simulink model "MemMgrForClima"  (R2022b)
settings: Solver=FixedStepDiscrete  StartTime=0.0  StopTime=10.0  FixedStep=0.1  RelTol=1e-3
model workspace (MAT data in the model file):
  MemMgrForClimaNvArray = Simulink.Signal
  MemMgrForClimaNvArray.DataType = 'UInt8'
  MemMgrForClimaNvArray.Dimensions = [254 1]
  MemMgrForClimaNvArray.DimensionsMode = 'auto'
  MemMgrForClimaNvArray.Complexity = 'real'
  MemMgrForClimaNvArray.SampleTime = -1
  MemMgrForClimaNvArray.SamplingMode = 'auto'
  MemMgrForClimaNvArray.InitialValue = 'MemMgrForClimaNvInit'
  MemMgrForClimaNvInit = Simulink.Parameter
  MemMgrForClimaNvInit.Value = [0; 0; 0; 0; 0; 0; 0; 0; 0; 0; 0; 0; 0; 0; 0; 0; 0; 0; 0; 0; 0; 0; 0; 0; 0; 0; 0; 0; 0; 0; 0; 0; 0; 0; 0; 0; 0; 0; 0; 0; 0; 0; 0; 0; 0; 0; 0; 0; 0; 0; 0; 0; 0; 0; 0; 0; 0; 0; 0; 0; 0; 0; 0; 0; 0; 0; 0; 0; 0; 0; 0; 0; 0; 0; 0; 0; 0; 0; 0; 0; 0; 0; 0; 0; 0; 0; 0; 0; 0; 0; 0; 0; 0; 0; 0; 0; 0; 0; 0; 0; 0; 0; 0; 0; 0; 0; 0; 0; 0; 0; 0; 0; 0; 0; 0; 0; 0; 0; 0; 0; 0; 0; 0; 0; 0; 0; 0; 0; 0; 0; 0; 0; 0; 0; 0; 0; 0; 0; 0; 0; 0; 0; 0; 0; 0; 0; 0; 0; 0; 0; 0; 0; 0; 0; 0; 0; 0; 0; 0; 0; 0; 0; 0; 0; 0; 0; 0; 0; 0; 0; 0; 0; 0; 0; 0; 0; 0; 0; 0; 0; 0; 0; 0; 0; 0; 0; 0; 0; 0; 0; 0; 0; 0; 0; 0; 0; 0; 0; 0; 0; 0; 0; 0; 0; 0; 0; 0; 0; 0; 0; 0; 0; 0; 0; 0; 0; 0; 0; 0; 0; 0; 0; 0; 0; 0; 0; 0; 0; 0; 0; 0; 0; 0; 0; 0; 0; 0; 0; 0; 0; 0; 0; 0; 0; 0; 0; 0; 0; 0; 0; 0; 0; 0; 0]
  MemMgrForClimaNvInit.DataType = 'UInt8'
  MemMgrForClimaNvInit.Complexity = 'real'
  MemMgrForClimaNvInit.Dimensions = [254 1]
(part 1 of 2)
settings Model: Creator=blue.ge  LastModifiedBy=blue.ge
settings DataIOCC: SaveFormat=StructureWithTime
settings OptimizationCC: DefaultParameterBehavior=Inlined  EnhancedBackFolding=on  PassReuseOutputArgsAs=Individual arguments  ZeroExternalMemoryAtStartup=off  ZeroInternalMemoryAtStartup=off  OptimizeBlockOrder=speed  DifferentSizesBufferReuse=on  OptimizationCustomize=off  EfficientTunableParamExpr=on
settings DebuggingCC: ParameterTunabilityLossMsg=error
settings ModelReferenceCC: ModelReferenceNumInstancesAllowed=Single
settings SFSimCC: MATLABDynamicMemAlloc=off
settings RTWCC: Description=AUTOSAR  SystemTargetFile=autosar.tlc  GenCodeOnly=on  TemplateMakefile=ert_default_tmf
settings CodeAppCC: ForceParamTrailComments=on  IgnoreCustomStorageClasses=off  OperatorAnnotations=on  SimulinkDataObjDesc=on  SFDataObjDesc=on  InsertBlockDesc=on
settings STFCustomTargetCC: TargetLangStandard=C99 (ISO)  MultiwordLength=256  ParMdlRefBuildCompliant=on  CompOptLevelCompliant=on  IncludeMdlTerminateFcn=off  CombineOutputUpdateFcns=on  SuppressErrorStatus=on  ERTCustomFileBanners=on  SupportNonFinite=off  SupportComplex=off  SupportContinuousTime=off  SupportNonInlinedSFcns=off  RemoveResetFunc=on  MATLABClassNameForMDSCustomization=Simulink.SoftwareTarget.ERTCustomization  AutosarCompliant=on  UseToolchainInfoCompliant=on  LookupTableObjectStructAxisOrder=2,1,3,4,...  LUTObjectStructOrderEvenSpacing=Size,Table,Breakpoints  SystemTargetFile=autosar.tlc
settings ERTTargetCC: TargetLangStandard=C99 (ISO)  ConcurrentExecutionCompliant=off  IncludeMdlTerminateFcn=off  SuppressErrorStatus=on  SupportNonFinite=off  SupportComplex=off  ModelStepFunctionPrototypeControlCompliant=off  CPPClassGenCompliant=off  AutosarCompliant=on  LookupTableObjectStructAxisOrder=2,1,3,4,...  LUTObjectStructOrderEvenSpacing=Size,Table,Breakpoints  InlinedParameterPlacement=NonHierarchical  ERTCustomFileTemplate=example_file_process.tlc

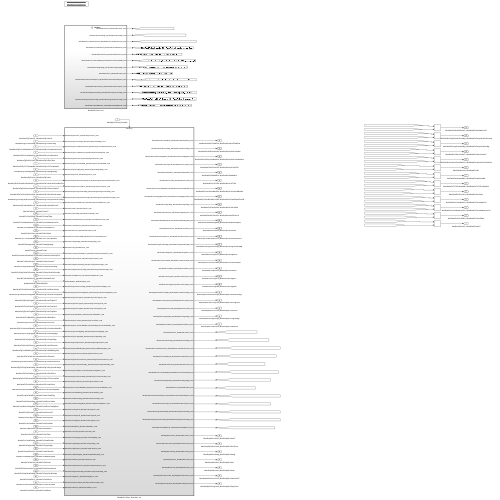
[thumbnail: Simulink's stored preview of the top-level diagram]
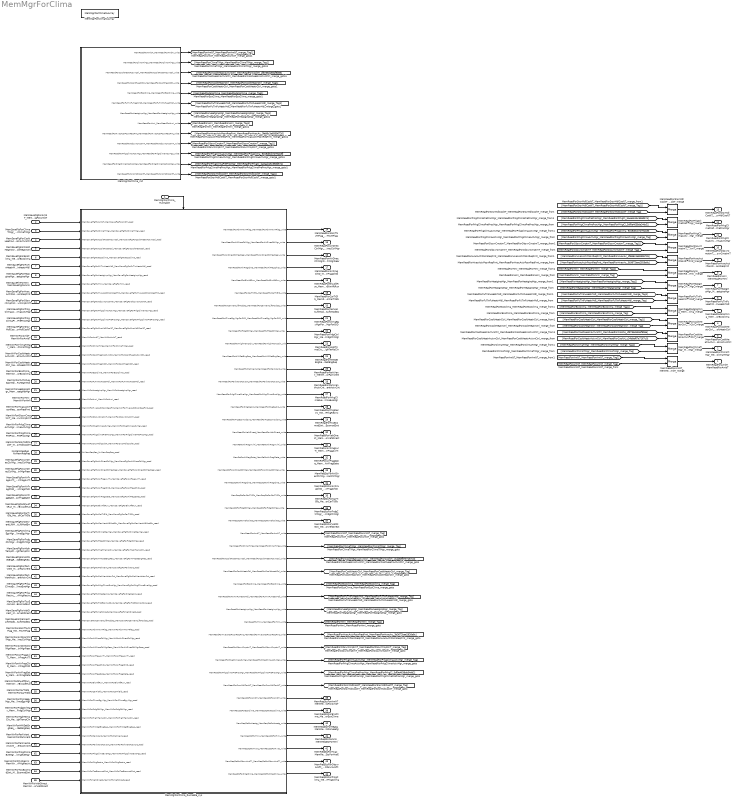
== system MemMgrForClima ==
  MemMgrForClima_Runnable : Inport  SampleTime=0.1  OutputFunctionCall=on
  MemSaveFlgForAmbT_MemSaveFlgForAmbT : Inport  Port=2  OutDataTypeStr=Boolean  PortDimensions=1
  MemSaveFlgForClimaTiMgr_MemSaveFlgForClimaTiMgr : Inport  Port=3  OutDataTypeStr=Boolean  PortDimensions=1
  MemSaveFlgForCooltHeatrActvnCtrl_MemSaveFlgForCooltHeatrActvnCtrl : Inport  Port=4  OutDataTypeStr=Boolean  PortDimensions=1
  MemSaveFlgForCooltHeatrCtrl_MemSaveFlgForCooltHeatrCtrl : Inport  Port=5  OutDataTypeStr=Boolean  PortDimensions=1
  MemSaveFlgForEcoClima_MemSaveFlgForEcoClima : Inport  Port=6  OutDataTypeStr=Boolean  PortDimensions=1
  MemSaveFlgForFuTInHeatrMdl_MemSaveFlgForFuTInHeatrMdl : Inport  Port=7  OutDataTypeStr=Boolean  PortDimensions=1
  MemSaveFlgForHeatgInpMgr_MemSaveFlgForHeatgInpMgr : Inport  Port=8  OutDataTypeStr=Boolean  PortDimensions=1
  MemSaveFlgForHmi_MemSaveFlgForHmi : Inport  Port=9  OutDataTypeStr=Boolean  PortDimensions=1
  MemSaveFlgForHvacActrPosnReqFrnt_MemSaveFlgForHvacActrPosnReqFrnt : Inport  Port=10  OutDataTypeStr=Boolean  PortDimensions=1
  MemSaveFlgForObsvrCmptmtT_MemSaveFlgForObsvrCmptmtT : Inport  Port=11  OutDataTypeStr=Boolean  PortDimensions=1
  MemSaveFlgForPrkgClimaActvMgr_MemSaveFlgForPrkgClimaActvMgr : Inport  Port=12  OutDataTypeStr=Boolean  PortDimensions=1
  MemSaveFlgForPrkgClimaPreProcMgr_MemSaveFlgForPrkgClimaPreProcMgr : Inport  Port=13  OutDataTypeStr=Boolean  PortDimensions=1
  MemSaveFlgForSnsrMdlCooltT_MemSaveFlgForSnsrMdlCooltT : Inport  Port=14  OutDataTypeStr=Boolean  PortDimensions=1
  MemWrForAmbT_MemWrForAmbT : Inport  Port=15  OutDataTypeStr=T30  PortDimensions=1
  MemWrForClimaTiMgr_MemWrForClimaTiMgr : Inport  Port=16  OutDataTypeStr=Bus: MemRecForClimaTiMgr  PortDimensions=1  BusOutputAsStruct=on
  MemWrForCooltHeatrActvnCtrl_MemWrForCooltHeatrActvnCtrl : Inport  Port=17  OutDataTypeStr=Bus: MemRecForCooltHeatrActvnCtrl  PortDimensions=1  BusOutputAsStruct=on
  MemWrForCooltHeatrCtrl_MemWrForCooltHeatrCtrl : Inport  Port=18  OutDataTypeStr=Bus: MemRecForCooltHeatrCtrl  PortDimensions=1  BusOutputAsStruct=on
  MemWrForEcoClima_MemWrForEcoClima : Inport  Port=19  OutDataTypeStr=Bus: MemWrForEcoClima  PortDimensions=1  BusOutputAsStruct=on
  MemWrForFuTInFuHeatrMdl_MemWrForFuTInFuHeatrMdl : Inport  Port=20  OutDataTypeStr=Bus: MemRecForFuTInFuHeatrMdl  PortDimensions=1  BusOutputAsStruct=on
  MemWrForHeatgInpMgr_MemWrForHeatgInpMgr : Inport  Port=21  OutDataTypeStr=Bus: MemRecForHeatgInpMgr  PortDimensions=1  BusOutputAsStruct=on
  MemWrForHmi_MemWrForHmi : Inport  Port=22  OutDataTypeStr=Bus: MemRecForHmi  PortDimensions=1  BusOutputAsStruct=on
  MemWrForHvacActrPosnReqFrnt_MemWrForHvacActrPosnReqFrnt : Inport  Port=23  OutDataTypeStr=Bus: MemRecForHvacActrPosnReqFrnt  PortDimensions=1  BusOutputAsStruct=on
  MemWrForObsvrCmptmT_MemWrForObsvrCmptmT : Inport  Port=24  OutDataTypeStr=Bus: MemRecForObsvrCmptmT  PortDimensions=1  BusOutputAsStruct=on
  MemWrForPrkgClimaActvMgr_MemWrForPrkgClimaActvMgr : Inport  Port=25  OutDataTypeStr=Bus: MemRecForPrkgClimaActvMgr  PortDimensions=1  BusOutputAsStruct=on
  MemWrForPrkgClimaPreProcMgr_MemWrForPrkgClimaPreProcMgr : Inport  Port=26  OutDataTypeStr=Bus: MemRecForPrkgClimaPreProcMgr  PortDimensions=1  BusOutputAsStruct=on
  MemWrForSnsrMdlCooltT_MemWrForSnsrMdlCooltT : Inport  Port=27  OutDataTypeStr=Bus: MemRecForSnsrMdlCooltT  PortDimensions=1  BusOutputAsStruct=on
  NvMemReqRes_NvMemReqRes : Inport  Port=28  OutDataTypeStr=Enum: NvMemReqRes  PortDimensions=1
  MemSaveFlgForAirDirecCtrlMgr_MemSaveFlgForAirDirecCtrlMgr : Inport  Port=29  OutDataTypeStr=Boolean  PortDimensions=1
  MemSaveFlgForAirDirecCtrlMgrRear_MemSaveFlgForAirDirecCtrlMgrRear : Inport  Port=30  OutDataTypeStr=Boolean  PortDimensions=1
  MemSaveFlgForAirFragAvlTi_MemSaveFlgForAirFragAvlTi : Inport  Port=31  OutDataTypeStr=Boolean  PortDimensions=1
  MemSaveFlgForAirFragChID_MemSaveFlgForAirFragChID : Inport  Port=32  OutDataTypeStr=Boolean  PortDimensions=1
  MemSaveFlgForAirFragDate_MemSaveFlgForAirFragDate : Inport  Port=33  OutDataTypeStr=Boolean  PortDimensions=1
  MemSaveFlgForBlwAftRun_MemSaveFlgForBlwAftRun : Inport  Port=34  OutDataTypeStr=Boolean  PortDimensions=1
  MemSaveFlgForCarTiGlb_MemSaveFlgForCarTiGlb : Inport  Port=35  OutDataTypeStr=Boolean  PortDimensions=1
  MemSaveFlgForCenVentLiftModErr_MemSaveFlgForCenVentLiftModErr : Inport  Port=36  OutDataTypeStr=Boolean  PortDimensions=1
  MemSaveFlgForClimaEgyMgr_MemSaveFlgForClimaEgyMgr : Inport  Port=37  OutDataTypeStr=Boolean  PortDimensions=1
  MemSaveFlgForFrdgCtrlMgr_MemSaveFlgForFrdgCtrlMgr : Inport  Port=38  OutDataTypeStr=Boolean  PortDimensions=1
  MemSaveFlgForHighTempCln_MemSaveFlgForHighTempCln : Inport  Port=39  OutDataTypeStr=Boolean  PortDimensions=1
  MemSaveFlgForHMISetEngReq_MemSaveFlgForHMISetEngReq : Inport  Port=40  OutDataTypeStr=Boolean  PortDimensions=1
  MemSaveFlgForParkVent_MemSaveFlgForParkVent : Inport  Port=41  OutDataTypeStr=Boolean  PortDimensions=1
  MemSaveFlgForParkVentActvCnt_MemSaveFlgForParkVentActvCnt : Inport  Port=42  OutDataTypeStr=Boolean  PortDimensions=1
  MemSaveFlgForPrkgClimaEveMgr_MemSaveFlgForPrkgClimaEveMgr : Inport  Port=43  OutDataTypeStr=Boolean  PortDimensions=1
  MemSaveFlgForPrkgRecirc_MemSaveFlgForPrkgRecirc : Inport  Port=44  OutDataTypeStr=Boolean  PortDimensions=1
  MemSaveFlgForTcsEcomodSwt_MemSaveFlgForTcsEcomodSwt : Inport  Port=45  OutDataTypeStr=Boolean  PortDimensions=1
  MemSaveFlgForVehDirect_MemSaveFlgForVehDirect : Inport  Port=46  OutDataTypeStr=Boolean  PortDimensions=1
  MemSaveForCenVentLiftModSts_MemSaveForCenVentLiftModSts : Inport  Port=47  OutDataTypeStr=Bus: MemSaveLiftMod  PortDimensions=1  BusOutputAsStruct=on
  MemWrForAbmTNvMFlag_MemWrForAbmTNvMFlag : Inport  Port=48  OutDataTypeStr=Boolean  PortDimensions=1
  MemWrForAirDirecCtrlMgr_MemWrForAirDirecCtrlMgr : Inport  Port=49  OutDataTypeStr=Bus: MemRecForAirDirecCtrlMgr  PortDimensions=1  BusOutputAsStruct=on
  MemWrForAirDirecCtrlMgrRear_MemWrForAirDirecCtrlMgrRear : Inport  Port=50  OutDataTypeStr=Bus: MemRecForAirDirecCtrlMgrRear  PortDimensions=1  BusOutputAsStruct=on
  MemWrForAirFragAvlTi_MemWrForAirFragAvlTi : Inport  Port=51  OutDataTypeStr=Bus: MemReqForAirFragAvlTi  PortDimensions=1  BusOutputAsStruct=on
  MemWrForAirFragChID_MemWrForAirFragChID : Inport  Port=52  OutDataTypeStr=Bus: MemReqForAirFragChID  PortDimensions=1  BusOutputAsStruct=on
  MemWrForAirFragDate_MemWrForAirFragDate : Inport  Port=53  OutDataTypeStr=Bus: MemWrForAirFragDate  PortDimensions=1  BusOutputAsStruct=on
  MemWrForBlwAftRun_MemWrForBlwAftRun : Inport  Port=54  OutDataTypeStr=Bus: MemRecForBlwAftRun  PortDimensions=1  BusOutputAsStruct=on
  MemWrForCarTiGlb_MemWrForCarTiGlb : Inport  Port=55  OutDataTypeStr=CarTiGlb  PortDimensions=1
  MemWrForClimaEgyMgr_MemWrForClimaEgyMgr : Inport  Port=56  OutDataTypeStr=Bus: MemRecForClimaEgyMgr  PortDimensions=1  BusOutputAsStruct=on
  MemWrForFrdgCtrlMgr_MemWrForFrdgCtrlMgr : Inport  Port=57  OutDataTypeStr=Bus: MemRecForFrdgCtrlMgr  PortDimensions=1  BusOutputAsStruct=on
  MemWrForHighTempCln_MemWrForHighTempCln : Inport  Port=58  OutDataTypeStr=Bus: MemRecForHighTempCln  PortDimensions=1  BusOutputAsStruct=on
  MemWrForHMISetEngReq_MemWrForHMISetEngReq : Inport  Port=59  OutDataTypeStr=Bus: MemRecForHMISetEngReq  PortDimensions=1  BusOutputAsStruct=on
  MemWrForParkVent_MemWrForParkVent : Inport  Port=60  OutDataTypeStr=Bus: MemRecForParkVent  PortDimensions=1  BusOutputAsStruct=on
  MemWrForParkVentActvCnt_MemWrForParkVentActvCnt : Inport  Port=61  OutDataTypeStr=Bus: MemRecForParkVentCnt  PortDimensions=1  BusOutputAsStruct=on
  MemWrForPrkgClimaEveMgr_MemWrForPrkgClimaEveMgr : Inport  Port=62  OutDataTypeStr=Bus: MemRecForPrkgClimaEveMgr  PortDimensions=1  BusOutputAsStruct=on
  MemWrForPrkgRecirc_MemWrForPrkgRecirc : Inport  Port=63  OutDataTypeStr=UInt8  PortDimensions=1
  MemWrForTcsEcomodSwt_MemWrForTcsEcomodSwt : Inport  Port=64  OutDataTypeStr=Boolean  PortDimensions=1
  MemWrForVehDirect_MemWrForVehDirect : Inport  Port=65  OutDataTypeStr=VCCVehDirect  PortDimensions=1
  MemMgrForClimaNvArray : DataStoreMemory  DataStoreName=MemMgrForClimaNvArray  InitialValue=MemMgrForClimaNvInit  ShowAdditionalParam=off  Dimensions=254  StateMustResolveToSignalObject=on
  MemMgrForClima_Init : Subsystem
  MemMgrForClima_Runnable_sys : Subsystem
  MemReadForAmbT_MemReadForAmbT_merge : Merge
  MemReadForClimaTiMgr_MemReadForClimaTiMgr_merge : Merge
  MemReadForCooltHeatrActvnCtrl_MemReadForCooltHeatrActvnCtrl_merge : Merge
  MemReadForCooltHeatrCtrl_MemReadForCooltHeatrCtrl_merge : Merge
  MemReadForEcoClima_MemReadForEcoClima_merge : Merge
  MemReadForFuTInFuHeatrMdl_MemReadForFuTInFuHeatrMdl_merge : Merge
  MemReadForHeatgInpMgr_MemReadForHeatgInpMgr_merge : Merge
  MemReadForHmi_MemReadForHmi_merge : Merge
  MemReadForHvacActrPosnReqFrnt_MemReadForHvacActrPosnReqFrnt_merge : Merge
  MemReadForObsvrCmptmT_MemReadForObsvrCmptmT_merge : Merge
  MemReadForPrkgClimaActvMgr_MemReadForPrkgClimaActvMgr_merge : Merge
  MemReadForPrkgClimaPreProcMgr_MemReadForPrkgClimaPreProcMgr_merge : Merge
  MemReadForSnsrMdlCooltT_MemReadForSnsrMdlCooltT_merge : Merge
  MemReadForAmbT_MemReadForAmbT : Outport  OutDataTypeStr=T30  PortDimensions=1
  MemReadForClimaTiMgr_MemReadForClimaTiMgr : Outport  Port=2  OutDataTypeStr=Bus: MemRecForClimaTiMgr  PortDimensions=1  BusOutputAsStruct=on
  MemReadForCooltHeatrActvnCtrl_MemReadForCooltHeatrActvnCtrl : Outport  Port=3  OutDataTypeStr=Bus: MemRecForCooltHeatrActvnCtrl  PortDimensions=1  BusOutputAsStruct=on
  MemReadForCooltHeatrCtrl_MemReadForCooltHeatrCtrl : Outport  Port=4  OutDataTypeStr=Bus: MemRecForCooltHeatrCtrl  PortDimensions=1  BusOutputAsStruct=on
  MemReadForEcoClima_MemReadForEcoClima : Outport  Port=5  OutDataTypeStr=Bus: MemWrForEcoClima  PortDimensions=1  BusOutputAsStruct=on
  MemReadForFuTInFuHeatrMdl_MemReadForFuTInFuHeatrMdl : Outport  Port=6  OutDataTypeStr=Bus: MemRecForFuTInFuHeatrMdl  PortDimensions=1  BusOutputAsStruct=on
  MemReadForHeatgInpMgr_MemReadForHeatgInpMgr : Outport  Port=7  OutDataTypeStr=Bus: MemRecForHeatgInpMgr  PortDimensions=1  BusOutputAsStruct=on
  MemReadForHmi_MemReadForHmi : Outport  Port=8  OutDataTypeStr=Bus: MemRecForHmi  PortDimensions=1  BusOutputAsStruct=on
  MemReadForHvacActrPosnReqFrnt_MemReadForHvacActrPosnReqFrnt : Outport  Port=9  OutDataTypeStr=Bus: MemRecForHvacActrPosnReqFrnt  PortDimensions=1  BusOutputAsStruct=on
  MemReadForObsvrCmptmT_MemReadForObsvrCmptmT : Outport  Port=10  OutDataTypeStr=Bus: MemRecForObsvrCmptmT  PortDimensions=1  BusOutputAsStruct=on
  MemReadForPrkgClimaActvMgr_MemReadForPrkgClimaActvMgr : Outport  Port=11  OutDataTypeStr=Bus: MemRecForPrkgClimaActvMgr  PortDimensions=1  BusOutputAsStruct=on
  MemReadForPrkgClimaPreProcMgr_MemReadForPrkgClimaPreProcMgr : Outport  Port=12  OutDataTypeStr=Bus: MemRecForPrkgClimaPreProcMgr  PortDimensions=1  BusOutputAsStruct=on
  MemReadForSnsrMdlCooltT_MemReadForSnsrMdlCooltT : Outport  Port=13  OutDataTypeStr=Bus: MemRecForSnsrMdlCooltT  PortDimensions=1  BusOutputAsStruct=on
  MemReadForAbmTNvMFlag_MemReadForAbmTNvMFlag : Outport  Port=14  OutDataTypeStr=Boolean  PortDimensions=1
  MemReadForAirDirecCtrlMgr_MemReadForAirDirecCtrlMgr : Outport  Port=15  OutDataTypeStr=Bus: MemRecForAirDirecCtrlMgr  PortDimensions=1  BusOutputAsStruct=on
  MemReadForAirDirecCtrlMgrRear_MemReadForAirDirecCtrlMgrRear : Outport  Port=16  OutDataTypeStr=Bus: MemRecForAirDirecCtrlMgrRear  PortDimensions=1  BusOutputAsStruct=on
  MemReadForAirFragChID_MemReadForAirFragChID : Outport  Port=17  OutDataTypeStr=Bus: MemReqForAirFragChID  PortDimensions=1  BusOutputAsStruct=on
  MemReadForBlwAftRun_MemReadForBlwAftRun : Outport  Port=18  OutDataTypeStr=Bus: MemRecForBlwAftRun  PortDimensions=1  BusOutputAsStruct=on
  MemReadForCarTiGlb_MemReadForCarTiGlb : Outport  Port=19  OutDataTypeStr=CarTiGlb  PortDimensions=1
  MemReadForCenVentLiftModSts_MemReadForCenVentLiftModSts : Outport  Port=20  OutDataTypeStr=Bus: MemSaveLiftMod  PortDimensions=1  BusOutputAsStruct=on
  MemReadForClimaEgyMgrForDID_MemReadForClimaEgyMgrForDID : Outport  Port=21  OutDataTypeStr=Bus: MemRecForClimaEgyMgr  PortDimensions=1  BusOutputAsStruct=on
  MemReadForFrdgCtrlMgr_MemReadForFrdgCtrlMgr : Outport  Port=22  OutDataTypeStr=Bus: MemRecForFrdgCtrlMgr  PortDimensions=1  BusOutputAsStruct=on
  MemReadForHighTempCln_MemReadForHighTempCln : Outport  Port=23  OutDataTypeStr=Bus: MemRecForHighTempCln  PortDimensions=1  BusOutputAsStruct=on
  MemReadForHMISetEngReq_MemReadForHMISetEngReq : Outport  Port=24  OutDataTypeStr=Bus: MemRecForHMISetEngReq  PortDimensions=1  BusOutputAsStruct=on
  MemReadForParkVent_MemReadForParkVent : Outport  Port=25  OutDataTypeStr=Bus: MemRecForParkVent  PortDimensions=1  BusOutputAsStruct=on
  MemReadForParkVentActvCnt_MemReadForParkVentActvCnt : Outport  Port=26  OutDataTypeStr=Bus: MemRecForParkVentCnt  PortDimensions=1  BusOutputAsStruct=on
  MemReadForPrkgClimaEveMgr_MemReadForPrkgClimaEveMgr : Outport  Port=27  OutDataTypeStr=Bus: MemRecForPrkgClimaEveMgr  PortDimensions=1  BusOutputAsStruct=on
  MemReadForPrkgRecirc_MemReadForPrkgRecirc : Outport  Port=28  OutDataTypeStr=UInt8  PortDimensions=1
  MemReadForTcsEcomodSwt_MemReadForTcsEcomodSwt : Outport  Port=29  OutDataTypeStr=Boolean  PortDimensions=1
  MemReadForVehDirect_MemReadForVehDirect : Outport  Port=30  OutDataTypeStr=VCCVehDirect  PortDimensions=1
  MemReForAirFragAvlTi_MemReForAirFragAvlTi : Outport  Port=31  OutDataTypeStr=Bus: MemReqForAirFragAvlTi  PortDimensions=1  BusOutputAsStruct=on
  MemReForAirFragDate_MemReForAirFragDate : Outport  Port=32  OutDataTypeStr=Bus: MemWrForAirFragDate  PortDimensions=1  BusOutputAsStruct=on
  MemReqStsForAirDirecCtrlMgr_MemReqStsForAirDirecCtrlMgr : Outport  Port=33  OutDataTypeStr=Enum: MemReqSts  PortDimensions=1
  MemReqStsForAirFragChID_MemReqStsForAirFragChID : Outport  Port=34  OutDataTypeStr=Enum: MemReqSts  PortDimensions=1
  MemReqStsForCarTiGlb_MemReqStsForCarTiGlb : Outport  Port=35  OutDataTypeStr=Enum: MemReqSts  PortDimensions=1
  MemReqStsForFrdgCtrlMgr_MemReqStsForFrdgCtrlMgr : Outport  Port=36  OutDataTypeStr=Enum: MemReqSts  PortDimensions=1
  MemReqStsForVehDirect_MemReqStsForVehDirect : Outport  Port=37  OutDataTypeStr=Enum: MemReqSts  PortDimensions=1
  MemReqStsForAmbT_MemReqStsForAmbT : Outport  Port=38  OutDataTypeStr=Enum: MemReqSts  PortDimensions=1
  MemReqStsForEcoClima_MemReqStsForEcoClima : Outport  Port=39  OutDataTypeStr=Enum: MemReqSts  PortDimensions=1
  MemReqStsForHeatg_MemReqStsForHeatg : Outport  Port=40  OutDataTypeStr=Enum: MemReqSts  PortDimensions=1
  MemReqStsForHmi_MemReqStsForHmi : Outport  Port=41  OutDataTypeStr=Enum: MemReqSts  PortDimensions=1
  MemReqStsForHvac_MemReqStsForHvac : Outport  Port=42  OutDataTypeStr=Enum: MemReqSts  PortDimensions=1
  MemReqStsForObsvrAndTi_MemReqStsForObsvrAndTi : Outport  Port=43  OutDataTypeStr=Enum: MemReqSts  PortDimensions=1
  MemReqStsForPrkgClima_MemReqStsForPrkgClima : Outport  Port=44  OutDataTypeStr=Enum: MemReqSts  PortDimensions=1
  wires:
  MemSaveFlgForAmbT_MemSaveFlgForAmbT/1 -> MemMgrForClima_Runnable_sys/1(MemSaveFlgForAmbT_MemSaveFlgForAmbT_read)
  MemSaveFlgForClimaTiMgr_MemSaveFlgForClimaTiMgr/1 -> MemMgrForClima_Runnable_sys/2(MemSaveFlgForClimaTiMgr_MemSaveFlgForClimaTiMgr_read)
  MemSaveFlgForCooltHeatrActvnCtrl_MemSaveFlgForCooltHeatrActvnCtrl/1 -> MemMgrForClima_Runnable_sys/3(MemSaveFlgForCooltHeatrActvnCtrl_MemSaveFlgForCooltHeatrActvnCtrl_read)
  MemSaveFlgForCooltHeatrCtrl_MemSaveFlgForCooltHeatrCtrl/1 -> MemMgrForClima_Runnable_sys/4(MemSaveFlgForCooltHeatrCtrl_MemSaveFlgForCooltHeatrCtrl_read)
  MemSaveFlgForEcoClima_MemSaveFlgForEcoClima/1 -> MemMgrForClima_Runnable_sys/5(MemSaveFlgForEcoClima_MemSaveFlgForEcoClima_read)
  MemSaveFlgForFuTInHeatrMdl_MemSaveFlgForFuTInHeatrMdl/1 -> MemMgrForClima_Runnable_sys/6(MemSaveFlgForFuTInHeatrMdl_MemSaveFlgForFuTInHeatrMdl_read)
  MemSaveFlgForHeatgInpMgr_MemSaveFlgForHeatgInpMgr/1 -> MemMgrForClima_Runnable_sys/7(MemSaveFlgForHeatgInpMgr_MemSaveFlgForHeatgInpMgr_read)
  MemSaveFlgForHmi_MemSaveFlgForHmi/1 -> MemMgrForClima_Runnable_sys/8(MemSaveFlgForHmi_MemSaveFlgForHmi_read)
  MemSaveFlgForHvacActrPosnReqFrnt_MemSaveFlgForHvacActrPosnReqFrnt/1 -> MemMgrForClima_Runnable_sys/9(MemSaveFlgForHvacActrPosnReqFrnt_MemSaveFlgForHvacActrPosnReqFrnt_read)
  MemSaveFlgForObsvrCmptmtT_MemSaveFlgForObsvrCmptmtT/1 -> MemMgrForClima_Runnable_sys/10(MemSaveFlgForObsvrCmptmtT_MemSaveFlgForObsvrCmptmtT_read)
  MemSaveFlgForPrkgClimaActvMgr_MemSaveFlgForPrkgClimaActvMgr/1 -> MemMgrForClima_Runnable_sys/11(MemSaveFlgForPrkgClimaActvMgr_MemSaveFlgForPrkgClimaActvMgr_read)
  MemSaveFlgForPrkgClimaPreProcMgr_MemSaveFlgForPrkgClimaPreProcMgr/1 -> MemMgrForClima_Runnable_sys/12(MemSaveFlgForPrkgClimaPreProcMgr_MemSaveFlgForPrkgClimaPreProcMgr_read)
  MemSaveFlgForSnsrMdlCooltT_MemSaveFlgForSnsrMdlCooltT/1 -> MemMgrForClima_Runnable_sys/13(MemSaveFlgForSnsrMdlCooltT_MemSaveFlgForSnsrMdlCooltT_read)
  MemWrForAmbT_MemWrForAmbT/1 -> MemMgrForClima_Runnable_sys/14(MemWrForAmbT_MemWrForAmbT_read)
  MemWrForClimaTiMgr_MemWrForClimaTiMgr/1 -> MemMgrForClima_Runnable_sys/15(MemWrForClimaTiMgr_MemWrForClimaTiMgr_read)
  MemWrForCooltHeatrActvnCtrl_MemWrForCooltHeatrActvnCtrl/1 -> MemMgrForClima_Runnable_sys/16(MemWrForCooltHeatrActvnCtrl_MemWrForCooltHeatrActvnCtrl_read)
  MemWrForCooltHeatrCtrl_MemWrForCooltHeatrCtrl/1 -> MemMgrForClima_Runnable_sys/17(MemWrForCooltHeatrCtrl_MemWrForCooltHeatrCtrl_read)
  MemWrForEcoClima_MemWrForEcoClima/1 -> MemMgrForClima_Runnable_sys/18(MemWrForEcoClima_MemWrForEcoClima_read)
  MemWrForFuTInFuHeatrMdl_MemWrForFuTInFuHeatrMdl/1 -> MemMgrForClima_Runnable_sys/19(MemWrForFuTInFuHeatrMdl_MemWrForFuTInFuHeatrMdl_read)
  MemWrForHeatgInpMgr_MemWrForHeatgInpMgr/1 -> MemMgrForClima_Runnable_sys/20(MemWrForHeatgInpMgr_MemWrForHeatgInpMgr_read)
  MemWrForHmi_MemWrForHmi/1 -> MemMgrForClima_Runnable_sys/21(MemWrForHmi_MemWrForHmi_read)
  MemWrForHvacActrPosnReqFrnt_MemWrForHvacActrPosnReqFrnt/1 -> MemMgrForClima_Runnable_sys/22(MemWrForHvacActrPosnReqFrnt_MemWrForHvacActrPosnReqFrnt_read)
  MemWrForObsvrCmptmT_MemWrForObsvrCmptmT/1 -> MemMgrForClima_Runnable_sys/23(MemWrForObsvrCmptmT_MemWrForObsvrCmptmT_read)
  MemWrForPrkgClimaActvMgr_MemWrForPrkgClimaActvMgr/1 -> MemMgrForClima_Runnable_sys/24(MemWrForPrkgClimaActvMgr_MemWrForPrkgClimaActvMgr_read)
  MemWrForPrkgClimaPreProcMgr_MemWrForPrkgClimaPreProcMgr/1 -> MemMgrForClima_Runnable_sys/25(MemWrForPrkgClimaPreProcMgr_MemWrForPrkgClimaPreProcMgr_read)
  MemWrForSnsrMdlCooltT_MemWrForSnsrMdlCooltT/1 -> MemMgrForClima_Runnable_sys/26(MemWrForSnsrMdlCooltT_MemWrForSnsrMdlCooltT_read)
  NvMemReqRes_NvMemReqRes/1 -> MemMgrForClima_Runnable_sys/27(NvMemReqRes_NvMemReqRes_read)
  MemSaveFlgForAirDirecCtrlMgr_MemSaveFlgForAirDirecCtrlMgr/1 -> MemMgrForClima_Runnable_sys/28(MemSaveFlgForAirDirecCtrlMgr_MemSaveFlgForAirDirecCtrlMgr_read)
  MemSaveFlgForAirDirecCtrlMgrRear_MemSaveFlgForAirDirecCtrlMgrRear/1 -> MemMgrForClima_Runnable_sys/29(MemSaveFlgForAirDirecCtrlMgrRear_MemSaveFlgForAirDirecCtrlMgrRear_read)
  MemSaveFlgForAirFragAvlTi_MemSaveFlgForAirFragAvlTi/1 -> MemMgrForClima_Runnable_sys/30(MemSaveFlgForAirFragAvlTi_MemSaveFlgForAirFragAvlTi_read)
  MemSaveFlgForAirFragChID_MemSaveFlgForAirFragChID/1 -> MemMgrForClima_Runnable_sys/31(MemSaveFlgForAirFragChID_MemSaveFlgForAirFragChID_read)
  MemSaveFlgForAirFragDate_MemSaveFlgForAirFragDate/1 -> MemMgrForClima_Runnable_sys/32(MemSaveFlgForAirFragDate_MemSaveFlgForAirFragDate_read)
  MemSaveFlgForBlwAftRun_MemSaveFlgForBlwAftRun/1 -> MemMgrForClima_Runnable_sys/33(MemSaveFlgForBlwAftRun_MemSaveFlgForBlwAftRun_read)
  MemSaveFlgForCarTiGlb_MemSaveFlgForCarTiGlb/1 -> MemMgrForClima_Runnable_sys/34(MemSaveFlgForCarTiGlb_MemSaveFlgForCarTiGlb_read)
  MemSaveFlgForCenVentLiftModErr_MemSaveFlgForCenVentLiftModErr/1 -> MemMgrForClima_Runnable_sys/35(MemSaveFlgForCenVentLiftModErr_MemSaveFlgForCenVentLiftModErr_read)
  MemSaveFlgForClimaEgyMgr_MemSaveFlgForClimaEgyMgr/1 -> MemMgrForClima_Runnable_sys/36(MemSaveFlgForClimaEgyMgr_MemSaveFlgForClimaEgyMgr_read)
  MemSaveFlgForFrdgCtrlMgr_MemSaveFlgForFrdgCtrlMgr/1 -> MemMgrForClima_Runnable_sys/37(MemSaveFlgForFrdgCtrlMgr_MemSaveFlgForFrdgCtrlMgr_read)
  MemSaveFlgForHighTempCln_MemSaveFlgForHighTempCln/1 -> MemMgrForClima_Runnable_sys/38(MemSaveFlgForHighTempCln_MemSaveFlgForHighTempCln_read)
  MemSaveFlgForHMISetEngReq_MemSaveFlgForHMISetEngReq/1 -> MemMgrForClima_Runnable_sys/39(MemSaveFlgForHMISetEngReq_MemSaveFlgForHMISetEngReq_read)
  MemSaveFlgForParkVent_MemSaveFlgForParkVent/1 -> MemMgrForClima_Runnable_sys/40(MemSaveFlgForParkVent_MemSaveFlgForParkVent_read)
  MemSaveFlgForParkVentActvCnt_MemSaveFlgForParkVentActvCnt/1 -> MemMgrForClima_Runnable_sys/41(MemSaveFlgForParkVentActvCnt_MemSaveFlgForParkVentActvCnt_read)
  MemSaveFlgForPrkgClimaEveMgr_MemSaveFlgForPrkgClimaEveMgr/1 -> MemMgrForClima_Runnable_sys/42(MemSaveFlgForPrkgClimaEveMgr_MemSaveFlgForPrkgClimaEveMgr_read)
  MemSaveFlgForPrkgRecirc_MemSaveFlgForPrkgRecirc/1 -> MemMgrForClima_Runnable_sys/43(MemSaveFlgForPrkgRecirc_MemSaveFlgForPrkgRecirc_read)
  MemSaveFlgForTcsEcomodSwt_MemSaveFlgForTcsEcomodSwt/1 -> MemMgrForClima_Runnable_sys/44(MemSaveFlgForTcsEcomodSwt_MemSaveFlgForTcsEcomodSwt_read)
  MemSaveFlgForVehDirect_MemSaveFlgForVehDirect/1 -> MemMgrForClima_Runnable_sys/45(MemSaveFlgForVehDirect_MemSaveFlgForVehDirect_read)
  MemSaveForCenVentLiftModSts_MemSaveForCenVentLiftModSts/1 -> MemMgrForClima_Runnable_sys/46(MemSaveForCenVentLiftModSts_MemSaveForCenVentLiftModSts_read)
  MemWrForAbmTNvMFlag_MemWrForAbmTNvMFlag/1 -> MemMgrForClima_Runnable_sys/47(MemWrForAbmTNvMFlag_MemWrForAbmTNvMFlag_read)
  MemWrForAirDirecCtrlMgr_MemWrForAirDirecCtrlMgr/1 -> MemMgrForClima_Runnable_sys/48(MemWrForAirDirecCtrlMgr_MemWrForAirDirecCtrlMgr_read)
  MemWrForAirDirecCtrlMgrRear_MemWrForAirDirecCtrlMgrRear/1 -> MemMgrForClima_Runnable_sys/49(MemWrForAirDirecCtrlMgrRear_MemWrForAirDirecCtrlMgrRear_read)
  MemWrForAirFragAvlTi_MemWrForAirFragAvlTi/1 -> MemMgrForClima_Runnable_sys/50(MemWrForAirFragAvlTi_MemWrForAirFragAvlTi_read)
  MemWrForAirFragChID_MemWrForAirFragChID/1 -> MemMgrForClima_Runnable_sys/51(MemWrForAirFragChID_MemWrForAirFragChID_read)
  MemWrForAirFragDate_MemWrForAirFragDate/1 -> MemMgrForClima_Runnable_sys/52(MemWrForAirFragDate_MemWrForAirFragDate_read)
  MemWrForBlwAftRun_MemWrForBlwAftRun/1 -> MemMgrForClima_Runnable_sys/53(MemWrForBlwAftRun_MemWrForBlwAftRun_read)
  MemWrForCarTiGlb_MemWrForCarTiGlb/1 -> MemMgrForClima_Runnable_sys/54(MemWrForCarTiGlb_MemWrForCarTiGlb_read)
  MemWrForClimaEgyMgr_MemWrForClimaEgyMgr/1 -> MemMgrForClima_Runnable_sys/55(MemWrForClimaEgyMgr_MemWrForClimaEgyMgr_read)
  MemWrForFrdgCtrlMgr_MemWrForFrdgCtrlMgr/1 -> MemMgrForClima_Runnable_sys/56(MemWrForFrdgCtrlMgr_MemWrForFrdgCtrlMgr_read)
  MemWrForHighTempCln_MemWrForHighTempCln/1 -> MemMgrForClima_Runnable_sys/57(MemWrForHighTempCln_MemWrForHighTempCln_read)
  MemWrForHMISetEngReq_MemWrForHMISetEngReq/1 -> MemMgrForClima_Runnable_sys/58(MemWrForHMISetEngReq_MemWrForHMISetEngReq_read)
  MemWrForParkVent_MemWrForParkVent/1 -> MemMgrForClima_Runnable_sys/59(MemWrForParkVent_MemWrForParkVent_read)
  MemWrForParkVentActvCnt_MemWrForParkVentActvCnt/1 -> MemMgrForClima_Runnable_sys/60(MemWrForParkVentActvCnt_MemWrForParkVentActvCnt_read)
  MemWrForPrkgClimaEveMgr_MemWrForPrkgClimaEveMgr/1 -> MemMgrForClima_Runnable_sys/61(MemWrForPrkgClimaEveMgr_MemWrForPrkgClimaEveMgr_read)
  MemWrForPrkgRecirc_MemWrForPrkgRecirc/1 -> MemMgrForClima_Runnable_sys/62(MemWrForPrkgRecirc_MemWrForPrkgRecirc_read)
  MemWrForTcsEcomodSwt_MemWrForTcsEcomodSwt/1 -> MemMgrForClima_Runnable_sys/63(MemWrForTcsEcomodSwt_MemWrForTcsEcomodSwt_read)
  MemWrForVehDirect_MemWrForVehDirect/1 -> MemMgrForClima_Runnable_sys/64(MemWrForVehDirect_MemWrForVehDirect_read)
  MemMgrForClima_Runnable_sys/1(MemReadForAbmTNvMFlag_MemReadForAbmTNvMFlag_write) -> MemReadForAbmTNvMFlag_MemReadForAbmTNvMFlag/1
  MemMgrForClima_Runnable_sys/2(MemReadForAirDirecCtrlMgr_MemReadForAirDirecCtrlMgr_write) -> MemReadForAirDirecCtrlMgr_MemReadForAirDirecCtrlMgr/1
  MemMgrForClima_Runnable_sys/3(MemReadForAirDirecCtrlMgrRear_MemReadForAirDirecCtrlMgrRear_write) -> MemReadForAirDirecCtrlMgrRear_MemReadForAirDirecCtrlMgrRear/1
  MemMgrForClima_Runnable_sys/4(MemReadForAirFragChID_MemReadForAirFragChID_write) -> MemReadForAirFragChID_MemReadForAirFragChID/1
  MemMgrForClima_Runnable_sys/5(MemReadForBlwAftRun_MemReadForBlwAftRun_write) -> MemReadForBlwAftRun_MemReadForBlwAftRun/1
  MemMgrForClima_Runnable_sys/6(MemReadForCarTiGlb_MemReadForCarTiGlb_write) -> MemReadForCarTiGlb_MemReadForCarTiGlb/1
  MemMgrForClima_Runnable_sys/7(MemReadForCenVentLiftModSts_MemReadForCenVentLiftModSts_write) -> MemReadForCenVentLiftModSts_MemReadForCenVentLiftModSts/1
  MemMgrForClima_Runnable_sys/8(MemReadForClimaEgyMgrForDID_MemReadForClimaEgyMgrForDID_write) -> MemReadForClimaEgyMgrForDID_MemReadForClimaEgyMgrForDID/1
  MemMgrForClima_Runnable_sys/9(MemReadForFrdgCtrlMgr_MemReadForFrdgCtrlMgr_write) -> MemReadForFrdgCtrlMgr_MemReadForFrdgCtrlMgr/1
  MemMgrForClima_Runnable_sys/10(MemReadForHighTempCln_MemReadForHighTempCln_write) -> MemReadForHighTempCln_MemReadForHighTempCln/1
  MemMgrForClima_Runnable_sys/11(MemReadForHMISetEngReq_MemReadForHMISetEngReq_write) -> MemReadForHMISetEngReq_MemReadForHMISetEngReq/1
  MemMgrForClima_Runnable_sys/12(MemReadForParkVent_MemReadForParkVent_write) -> MemReadForParkVent_MemReadForParkVent/1
  MemMgrForClima_Runnable_sys/13(MemReadForParkVentActvCnt_MemReadForParkVentActvCnt_write) -> MemReadForParkVentActvCnt_MemReadForParkVentActvCnt/1
  MemMgrForClima_Runnable_sys/14(MemReadForPrkgClimaEveMgr_MemReadForPrkgClimaEveMgr_write) -> MemReadForPrkgClimaEveMgr_MemReadForPrkgClimaEveMgr/1
  MemMgrForClima_Runnable_sys/15(MemReadForPrkgRecirc_MemReadForPrkgRecirc_write) -> MemReadForPrkgRecirc_MemReadForPrkgRecirc/1
  MemMgrForClima_Runnable_sys/16(MemReadForTcsEcomodSwt_MemReadForTcsEcomodSwt_write) -> MemReadForTcsEcomodSwt_MemReadForTcsEcomodSwt/1
  MemMgrForClima_Runnable_sys/17(MemReadForVehDirect_MemReadForVehDirect_write) -> MemReadForVehDirect_MemReadForVehDirect/1
  MemMgrForClima_Runnable_sys/18(MemReForAirFragAvlTi_MemReForAirFragAvlTi_write) -> MemReForAirFragAvlTi_MemReForAirFragAvlTi/1
  MemMgrForClima_Runnable_sys/19(MemReForAirFragDate_MemReForAirFragDate_write) -> MemReForAirFragDate_MemReForAirFragDate/1
  MemMgrForClima_Runnable_sys/20(MemReqStsForAirDirecCtrlMgr_MemReqStsForAirDirecCtrlMgr_write) -> MemReqStsForAirDirecCtrlMgr_MemReqStsForAirDirecCtrlMgr/1
  MemMgrForClima_Runnable_sys/21(MemReqStsForAirFragChID_MemReqStsForAirFragChID_write) -> MemReqStsForAirFragChID_MemReqStsForAirFragChID/1
  MemMgrForClima_Runnable_sys/22(MemReqStsForCarTiGlb_MemReqStsForCarTiGlb_write) -> MemReqStsForCarTiGlb_MemReqStsForCarTiGlb/1
  MemMgrForClima_Runnable_sys/23(MemReqStsForFrdgCtrlMgr_MemReqStsForFrdgCtrlMgr_write) -> MemReqStsForFrdgCtrlMgr_MemReqStsForFrdgCtrlMgr/1
  MemMgrForClima_Runnable_sys/24(MemReqStsForVehDirect_MemReqStsForVehDirect_write) -> MemReqStsForVehDirect_MemReqStsForVehDirect/1
  MemReadForAmbT_MemReadForAmbT_merge/1 -> MemReadForAmbT_MemReadForAmbT/1
  MemReadForClimaTiMgr_MemReadForClimaTiMgr_merge/1 -> MemReadForClimaTiMgr_MemReadForClimaTiMgr/1
  MemReadForCooltHeatrActvnCtrl_MemReadForCooltHeatrActvnCtrl_merge/1 -> MemReadForCooltHeatrActvnCtrl_MemReadForCooltHeatrActvnCtrl/1
  MemReadForCooltHeatrCtrl_MemReadForCooltHeatrCtrl_merge/1 -> MemReadForCooltHeatrCtrl_MemReadForCooltHeatrCtrl/1
  MemReadForEcoClima_MemReadForEcoClima_merge/1 -> MemReadForEcoClima_MemReadForEcoClima/1
  MemReadForFuTInFuHeatrMdl_MemReadForFuTInFuHeatrMdl_merge/1 -> MemReadForFuTInFuHeatrMdl_MemReadForFuTInFuHeatrMdl/1
  MemReadForHeatgInpMgr_MemReadForHeatgInpMgr_merge/1 -> MemReadForHeatgInpMgr_MemReadForHeatgInpMgr/1
  MemReadForHmi_MemReadForHmi_merge/1 -> MemReadForHmi_MemReadForHmi/1
  MemReadForHvacActrPosnReqFrnt_MemReadForHvacActrPosnReqFrnt_merge/1 -> MemReadForHvacActrPosnReqFrnt_MemReadForHvacActrPosnReqFrnt/1
  MemReadForObsvrCmptmT_MemReadForObsvrCmptmT_merge/1 -> MemReadForObsvrCmptmT_MemReadForObsvrCmptmT/1
  MemReadForPrkgClimaActvMgr_MemReadForPrkgClimaActvMgr_merge/1 -> MemReadForPrkgClimaActvMgr_MemReadForPrkgClimaActvMgr/1
  MemReadForPrkgClimaPreProcMgr_MemReadForPrkgClimaPreProcMgr_merge/1 -> MemReadForPrkgClimaPreProcMgr_MemReadForPrkgClimaPreProcMgr/1
  MemReadForSnsrMdlCooltT_MemReadForSnsrMdlCooltT_merge/1 -> MemReadForSnsrMdlCooltT_MemReadForSnsrMdlCooltT/1
  MemMgrForClima_Runnable_sys/38(MemReqStsForAmbT_MemReqStsForAmbT_write) -> MemReqStsForAmbT_MemReqStsForAmbT/1
  MemMgrForClima_Runnable_sys/39(MemReqStsForEcoClima_MemReqStsForEcoClima_write) -> MemReqStsForEcoClima_MemReqStsForEcoClima/1
  MemMgrForClima_Runnable_sys/40(MemReqStsForHeatg_MemReqStsForHeatg_write) -> MemReqStsForHeatg_MemReqStsForHeatg/1
  MemMgrForClima_Runnable_sys/41(MemReqStsForHmi_MemReqStsForHmi_write) -> MemReqStsForHmi_MemReqStsForHmi/1
  MemMgrForClima_Runnable_sys/42(MemReqStsForHvac_MemReqStsForHvac_write) -> MemReqStsForHvac_MemReqStsForHvac/1
  MemMgrForClima_Runnable_sys/43(MemReqStsForObsvrAndTi_MemReqStsForObsvrAndTi_write) -> MemReqStsForObsvrAndTi_MemReqStsForObsvrAndTi/1
  MemMgrForClima_Runnable_sys/44(MemReqStsForPrkgClima_MemReqStsForPrkgClima_write) -> MemReqStsForPrkgClima_MemReqStsForPrkgClima/1
  MemMgrForClima_Runnable_sys/37(MemReadForSnsrMdlCooltT_MemReadForSnsrMdlCooltT_write) -> [MemReadForSnsrMdlCooltT_MemReadForSnsrMdlCooltT_merge_Tag]
  [MemReadForSnsrMdlCooltT_MemReadForSnsrMdlCooltT_merge_Tag] -> MemReadForSnsrMdlCooltT_MemReadForSnsrMdlCooltT_merge/2
  MemMgrForClima_Init/13(MemReadForSnsrMdlCooltT_MemReadForSnsrMdlCooltT_write) -> [MemReadForSnsrMdlCooltT_MemReadForSnsrMdlCooltT_merge_Tag1]
  [MemReadForSnsrMdlCooltT_MemReadForSnsrMdlCooltT_merge_Tag1] -> MemReadForSnsrMdlCooltT_MemReadForSnsrMdlCooltT_merge/1
  MemMgrForClima_Runnable_sys/36(MemReadForPrkgClimaPreProcMgr_MemReadForPrkgClimaPreProcMgr_write) -> [MemReadForPrkgClimaPreProcMgr_MemReadForPrkgCl_5df0e4638da34dd2]
  [MemReadForPrkgClimaPreProcMgr_MemReadForPrkgCl_5df0e4638da34dd2] -> MemReadForPrkgClimaPreProcMgr_MemReadForPrkgClimaPreProcMgr_merge/2
  MemMgrForClima_Init/12(MemReadForPrkgClimaPreProcMgr_MemReadForPrkgClimaPreProcMgr_write) -> [MemReadForPrkgClimaPreProcMgr_MemReadForPrkgCl_baeaab15c558d574]
  [MemReadForPrkgClimaPreProcMgr_MemReadForPrkgCl_baeaab15c558d574] -> MemReadForPrkgClimaPreProcMgr_MemReadForPrkgClimaPreProcMgr_merge/1
  MemMgrForClima_Runnable_sys/35(MemReadForPrkgClimaActvMgr_MemReadForPrkgClimaActvMgr_write) -> [MemReadForPrkgClimaActvMgr_MemReadForPrkgClimaActvMgr_merge_Tag]
  [MemReadForPrkgClimaActvMgr_MemReadForPrkgClimaActvMgr_merge_Tag] -> MemReadForPrkgClimaActvMgr_MemReadForPrkgClimaActvMgr_merge/2
  MemMgrForClima_Init/11(MemReadForPrkgClimaActvMgr_MemReadForPrkgClimaActvMgr_write) -> [MemReadForPrkgClimaActvMgr_MemReadForPrkgClima_9019decb7279118f]
  [MemReadForPrkgClimaActvMgr_MemReadForPrkgClima_9019decb7279118f] -> MemReadForPrkgClimaActvMgr_MemReadForPrkgClimaActvMgr_merge/1
  MemMgrForClima_Runnable_sys/34(MemReadForObsvrCmptmT_MemReadForObsvrCmptmT_write) -> [MemReadForObsvrCmptmT_MemReadForObsvrCmptmT_merge_Tag]
  [MemReadForObsvrCmptmT_MemReadForObsvrCmptmT_merge_Tag] -> MemReadForObsvrCmptmT_MemReadForObsvrCmptmT_merge/2
  MemMgrForClima_Init/10(MemReadForObsvrCmptmT_MemReadForObsvrCmptmT_write) -> [MemReadForObsvrCmptmT_MemReadForObsvrCmptmT_merge_Tag1]
  [MemReadForObsvrCmptmT_MemReadForObsvrCmptmT_merge_Tag1] -> MemReadForObsvrCmptmT_MemReadForObsvrCmptmT_merge/1
  MemMgrForClima_Runnable_sys/33(MemReadForHvacActrPosnReqFrnt_MemReadForHvacActrPosnReqFrnt_write) -> [MemReadForHvacActrPosnReqFrnt_MemReadForHvacAc_3b3672bae2926a8c]
  [MemReadForHvacActrPosnReqFrnt_MemReadForHvacAc_3b3672bae2926a8c] -> MemReadForHvacActrPosnReqFrnt_MemReadForHvacActrPosnReqFrnt_merge/2
  MemMgrForClima_Init/9(MemReadForHvacActrPosnReqFrnt_MemReadForHvacActrPosnReqFrnt_write) -> [MemReadForHvacActrPosnReqFrnt_MemReadForHvacAc_3fe86c3a69396726]
  [MemReadForHvacActrPosnReqFrnt_MemReadForHvacAc_3fe86c3a69396726] -> MemReadForHvacActrPosnReqFrnt_MemReadForHvacActrPosnReqFrnt_merge/1
  MemMgrForClima_Runnable_sys/32(MemReadForHmi_MemReadForHmi_write) -> [MemReadForHmi_MemReadForHmi_merge_Tag]
  [MemReadForHmi_MemReadForHmi_merge_Tag] -> MemReadForHmi_MemReadForHmi_merge/2
  MemMgrForClima_Init/8(MemReadForHmi_MemReadForHmi_write) -> [MemReadForHmi_MemReadForHmi_merge_Tag1]
  [MemReadForHmi_MemReadForHmi_merge_Tag1] -> MemReadForHmi_MemReadForHmi_merge/1
  MemMgrForClima_Runnable_sys/31(MemReadForHeatgInpMgr_MemReadForHeatgInpMgr_write) -> [MemReadForHeatgInpMgr_MemReadForHeatgInpMgr_merge_Tag]
  [MemReadForHeatgInpMgr_MemReadForHeatgInpMgr_merge_Tag] -> MemReadForHeatgInpMgr_MemReadForHeatgInpMgr_merge/2
  MemMgrForClima_Init/7(MemReadForHeatgInpMgr_MemReadForHeatgInpMgr_write) -> [MemReadForHeatgInpMgr_MemReadForHeatgInpMgr_merge_Tag1]
  [MemReadForHeatgInpMgr_MemReadForHeatgInpMgr_merge_Tag1] -> MemReadForHeatgInpMgr_MemReadForHeatgInpMgr_merge/1
  MemMgrForClima_Runnable_sys/30(MemReadForFuTInFuHeatrMdl_MemReadForFuTInFuHeatrMdl_write) -> [MemReadForFuTInFuHeatrMdl_MemReadForFuTInFuHeatrMdl_merge_Tag]
  [MemReadForFuTInFuHeatrMdl_MemReadForFuTInFuHeatrMdl_merge_Tag] -> MemReadForFuTInFuHeatrMdl_MemReadForFuTInFuHeatrMdl_merge/2
  MemMgrForClima_Init/6(MemReadForFuTInFuHeatrMdl_MemReadForFuTInFuHeatrMdl_write) -> [MemReadForFuTInFuHeatrMdl_MemReadForFuTInFuHeatrMdl_merge_Tag1]
  [MemReadForFuTInFuHeatrMdl_MemReadForFuTInFuHeatrMdl_merge_Tag1] -> MemReadForFuTInFuHeatrMdl_MemReadForFuTInFuHeatrMdl_merge/1
  MemMgrForClima_Runnable_sys/29(MemReadForEcoClima_MemReadForEcoClima_write) -> [MemReadForEcoClima_MemReadForEcoClima_merge_Tag]
  [MemReadForEcoClima_MemReadForEcoClima_merge_Tag] -> MemReadForEcoClima_MemReadForEcoClima_merge/2
  MemMgrForClima_Init/5(MemReadForEcoClima_MemReadForEcoClima_write) -> [MemReadForEcoClima_MemReadForEcoClima_merge_Tag1]
  [MemReadForEcoClima_MemReadForEcoClima_merge_Tag1] -> MemReadForEcoClima_MemReadForEcoClima_merge/1
  MemMgrForClima_Runnable_sys/28(MemReadForCooltHeatrCtrl_MemReadForCooltHeatrCtrl_write) -> [MemReadForCooltHeatrCtrl_MemReadForCooltHeatrCtrl_merge_Tag]
  [MemReadForCooltHeatrCtrl_MemReadForCooltHeatrCtrl_merge_Tag] -> MemReadForCooltHeatrCtrl_MemReadForCooltHeatrCtrl_merge/2
  MemMgrForClima_Init/4(MemReadForCooltHeatrCtrl_MemReadForCooltHeatrCtrl_write) -> [MemReadForCooltHeatrCtrl_MemReadForCooltHeatrCtrl_merge_Tag1]
  [MemReadForCooltHeatrCtrl_MemReadForCooltHeatrCtrl_merge_Tag1] -> MemReadForCooltHeatrCtrl_MemReadForCooltHeatrCtrl_merge/1
  MemMgrForClima_Runnable_sys/27(MemReadForCooltHeatrActvnCtrl_MemReadForCooltHeatrActvnCtrl_write) -> [MemReadForCooltHeatrActvnCtrl_MemReadForCooltH_c34b6ef67e7167c0]
  [MemReadForCooltHeatrActvnCtrl_MemReadForCooltH_c34b6ef67e7167c0] -> MemReadForCooltHeatrActvnCtrl_MemReadForCooltHeatrActvnCtrl_merge/2
  MemMgrForClima_Init/3(MemReadForCooltHeatrActvnCtrl_MemReadForCooltHeatrActvnCtrl_write) -> [MemReadForCooltHeatrActvnCtrl_MemReadForCooltH_4f8736d4594f9b9e]
  [MemReadForCooltHeatrActvnCtrl_MemReadForCooltH_4f8736d4594f9b9e] -> MemReadForCooltHeatrActvnCtrl_MemReadForCooltHeatrActvnCtrl_merge/1
  MemMgrForClima_Runnable_sys/26(MemReadForClimaTiMgr_MemReadForClimaTiMgr_write) -> [MemReadForClimaTiMgr_MemReadForClimaTiMgr_merge_Tag]
  [MemReadForClimaTiMgr_MemReadForClimaTiMgr_merge_Tag] -> MemReadForClimaTiMgr_MemReadForClimaTiMgr_merge/2
  MemMgrForClima_Init/2(MemReadForClimaTiMgr_MemReadForClimaTiMgr_write) -> [MemReadForClimaTiMgr_MemReadForClimaTiMgr_merge_Tag1]
  [MemReadForClimaTiMgr_MemReadForClimaTiMgr_merge_Tag1] -> MemReadForClimaTiMgr_MemReadForClimaTiMgr_merge/1
  MemMgrForClima_Runnable_sys/25(MemReadForAmbT_MemReadForAmbT_write) -> [MemReadForAmbT_MemReadForAmbT_merge_Tag]
  [MemReadForAmbT_MemReadForAmbT_merge_Tag] -> MemReadForAmbT_MemReadForAmbT_merge/2
  MemMgrForClima_Init/1(MemReadForAmbT_MemReadForAmbT_write) -> [MemReadForAmbT_MemReadForAmbT_merge_Tag1]
  [MemReadForAmbT_MemReadForAmbT_merge_Tag1] -> MemReadForAmbT_MemReadForAmbT_merge/1
  MemMgrForClima_Runnable/1 -> MemMgrForClima_Runnable_sys/Trigger

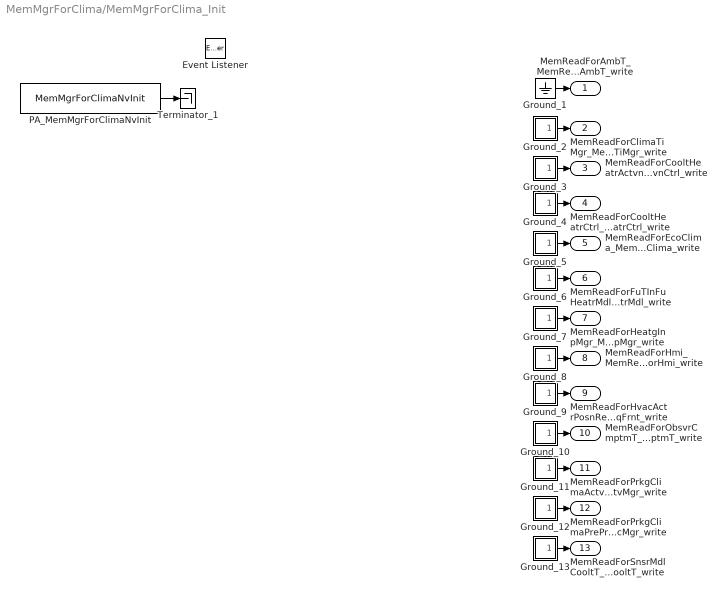
== system MemMgrForClima/MemMgrForClima_Init ==
  Event Listener : EventListener  EventName=reset
  Ground_1 : Ground
  Ground_10 : Subsystem
  Ground_11 : Subsystem
  Ground_12 : Subsystem
  Ground_13 : Subsystem
  Ground_2 : Subsystem
  Ground_3 : Subsystem
  Ground_4 : Subsystem
  Ground_5 : Subsystem
  Ground_6 : Subsystem
  Ground_7 : Subsystem
  Ground_8 : Subsystem
  Ground_9 : Subsystem
  PA_MemMgrForClimaNvInit : Constant  Value=MemMgrForClimaNvInit
  Terminator_1 : Terminator
  MemReadForAmbT_MemReadForAmbT_write : Outport
  MemReadForClimaTiMgr_MemReadForClimaTiMgr_write : Outport  Port=2
  MemReadForCooltHeatrActvnCtrl_MemReadForCooltHeatrActvnCtrl_write : Outport  Port=3
  MemReadForCooltHeatrCtrl_MemReadForCooltHeatrCtrl_write : Outport  Port=4
  MemReadForEcoClima_MemReadForEcoClima_write : Outport  Port=5
  MemReadForFuTInFuHeatrMdl_MemReadForFuTInFuHeatrMdl_write : Outport  Port=6
  MemReadForHeatgInpMgr_MemReadForHeatgInpMgr_write : Outport  Port=7
  MemReadForHmi_MemReadForHmi_write : Outport  Port=8
  MemReadForHvacActrPosnReqFrnt_MemReadForHvacActrPosnReqFrnt_write : Outport  Port=9
  MemReadForObsvrCmptmT_MemReadForObsvrCmptmT_write : Outport  Port=10
  MemReadForPrkgClimaActvMgr_MemReadForPrkgClimaActvMgr_write : Outport  Port=11
  MemReadForPrkgClimaPreProcMgr_MemReadForPrkgClimaPreProcMgr_write : Outport  Port=12
  MemReadForSnsrMdlCooltT_MemReadForSnsrMdlCooltT_write : Outport  Port=13
  wires:
  PA_MemMgrForClimaNvInit/1 -> Terminator_1/1
  Ground_1/1 -> MemReadForAmbT_MemReadForAmbT_write/1
  Ground_2/1(Out1) -> MemReadForClimaTiMgr_MemReadForClimaTiMgr_write/1
  Ground_3/1(Out1) -> MemReadForCooltHeatrActvnCtrl_MemReadForCooltHeatrActvnCtrl_write/1
  Ground_4/1(Out1) -> MemReadForCooltHeatrCtrl_MemReadForCooltHeatrCtrl_write/1
  Ground_5/1(Out1) -> MemReadForEcoClima_MemReadForEcoClima_write/1
  Ground_6/1(Out1) -> MemReadForFuTInFuHeatrMdl_MemReadForFuTInFuHeatrMdl_write/1
  Ground_7/1(Out1) -> MemReadForHeatgInpMgr_MemReadForHeatgInpMgr_write/1
  Ground_8/1(Out1) -> MemReadForHmi_MemReadForHmi_write/1
  Ground_9/1(Out1) -> MemReadForHvacActrPosnReqFrnt_MemReadForHvacActrPosnReqFrnt_write/1
  Ground_10/1(Out1) -> MemReadForObsvrCmptmT_MemReadForObsvrCmptmT_write/1
  Ground_11/1(Out1) -> MemReadForPrkgClimaActvMgr_MemReadForPrkgClimaActvMgr_write/1
  Ground_12/1(Out1) -> MemReadForPrkgClimaPreProcMgr_MemReadForPrkgClimaPreProcMgr_write/1
  Ground_13/1(Out1) -> MemReadForSnsrMdlCooltT_MemReadForSnsrMdlCooltT_write/1
